simulink model "CC_CarExample_ROS_template"  (R2022b)
settings: Solver=VariableStepAuto  StartTime=0.0  StopTime=100.0  RelTol=1e-3
model workspace (MAT data in the model file):
  Logfile_Data_Object : robotics.ros.BagSelection (value not decoded)
  Logfile_Path = '/Users/sprinkle/work/data/2023_09_28_16_19_25_2T3MWRFVXLW056972microstrain_record.bag'
  Logfile_Playback_Duration = nan
  Logfile_Playback_Offset = 110
libraries: robotlib x1
settings Model: Creator=sprinkle  LastModifiedBy=sprinkle
settings SolverCC: AlgebraicLoopSolver=TrustRegion
settings DebuggingCC: UnconnectedInputMsg=warning  UnconnectedOutputMsg=warning  UnconnectedLineMsg=warning  SFMachineParentedDataDiag=warning
settings ModelReferenceCC: EnableRefExpFcnMdlSchedulingChecks=on

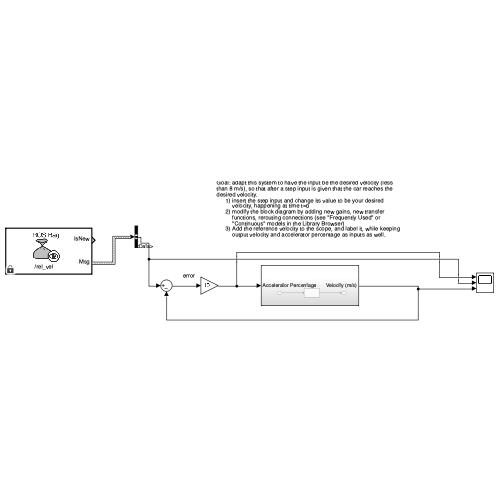
[thumbnail: Simulink's stored preview of the top-level diagram]
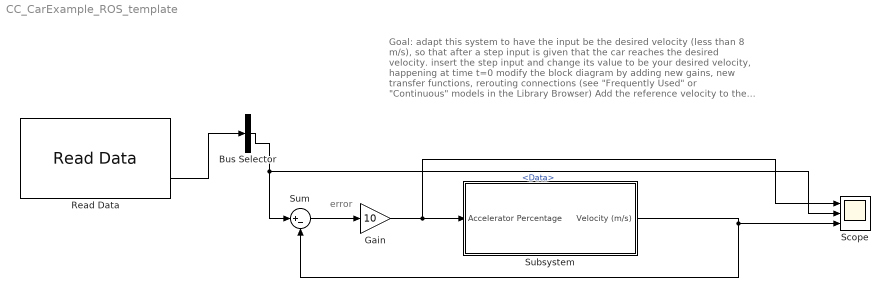
== system CC_CarExample_ROS_template ==
  note: error
  note: Goal: adapt this system to have the input be the desired velocity (less than 8 m/s), so that after a step input is given that the car reaches the desired velocity.
      | insert the step input and change its value to be your desired velocity, happening at time t=0
      | modify the block diagram by adding new gains, new transfer functions, rerouting connections (see "Frequently Used" or "Continuous" models in the Library Browser)
      | Add the reference velocity to the scope, and label it, while keeping output velocity and accelerator percentage as inputs as well.
  Bus Selector : BusSelector  OutputSignals=Data
  Gain : Gain  Gain=10
  Read Data : robotlib/Read Data  topic=/rel_vel  sampleTime=-1  logfileType=rosbag  modelName=CC_CarExample_ROS_template  msgType=std_msgs/Float64
      settings: LibrarySourceBlock=roslib/ROS/Read Data
  Scope : Scope  NumInputPorts=3
  Subsystem : Subsystem
  Sum : Sum  Inputs=|+-
  wires:
  Subsystem/1(Velocity (m/s)) -> Scope/3, Sum/2
  Bus Selector/1 -> Sum/1, Scope/2  "<Data>"
  Sum/1 -> Gain/1
  Gain/1 -> Scope/1, Subsystem/1(Accelerator Percentage)
  Read Data/2 -> Bus Selector/1

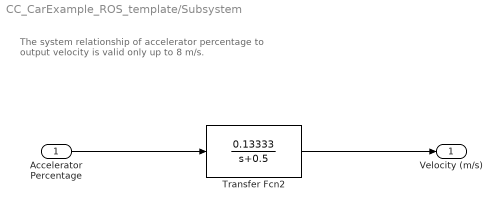
== system CC_CarExample_ROS_template/Subsystem ==
  note: The system relationship of accelerator percentage to output velocity is valid only up to 8 m/s.
  Accelerator Percentage : Inport
  Transfer Fcn2 : TransferFcn  Numerator=[0.13333]  Denominator=[1 0.5]
  Velocity (m/s) : Outport
  wires:
  Accelerator Percentage/1 -> Transfer Fcn2/1
  Transfer Fcn2/1 -> Velocity (m/s)/1
